simulink model "test_pfb_fft_full_full"  (R2013a)
settings: Solver=VariableStepDiscrete  StartTime=0.0  StopTime=1000  RelTol=1e-3
undefined symbols (not defined in the file): pfb_coeff_gen_calc
libraries: xbsIndex_r4 x21611, casper_library_misc x211, casper_library_delays x44, simulink x16, xbsReplacements_r4 x8, xps_library x1
(part 1 of 260)
settings Model: Creator=root  LastModifiedBy=root

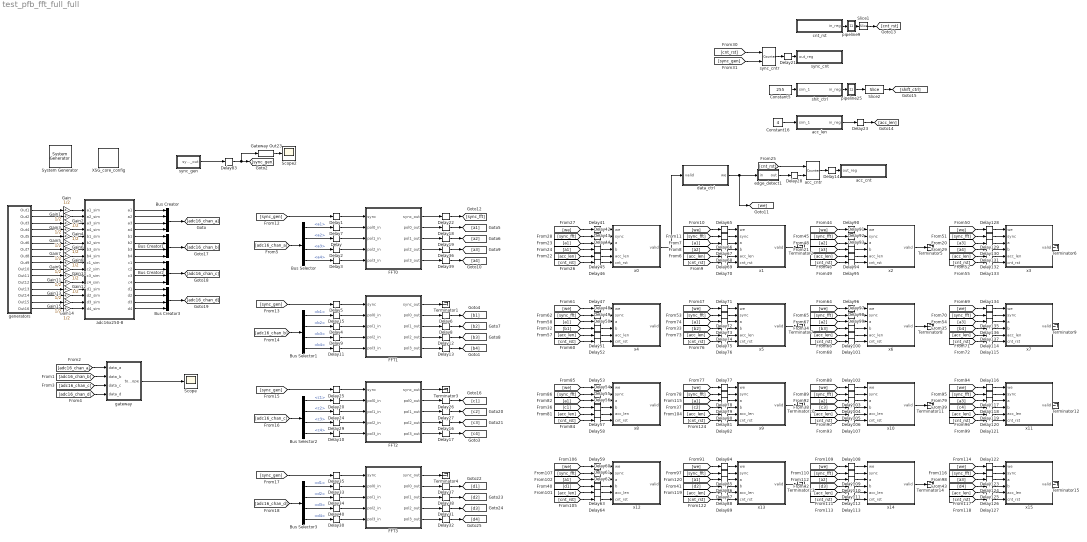
== system test_pfb_fft_full_full ==
  " System Generator" : xbsIndex_r4/ System Generator  Tag=genX  xilinxfamily=virtex6  part=xc6vsx475t  speed=-1  package=ff1759  synthesis_tool=XST  clock_wrapper=Clock Enables  directory=./test_pfb_fft_full_full/sysgen  proj_type=Project Navigator  Synth_file=XST Defaults  Impl_file=ISE Defaults  testbench=off  simulink_period=1  sysclk_period=5  dcm_input_clock_period=100  incr_netlist=off  trim_vbits=Everywhere in SubSystem  dbl_ovrd=According to Block Masks  core_generation=According to Block Masks  run_coregen=off  deprecated_control=off  eval_field=0  block_type=sysgen
  Bus Creator{,1..3} (4 alike) : BusCreator
  Bus Selector : BusSelector  OutputSignals=a1,a2,a3,a4
  Bus Selector1 : BusSelector  OutputSignals=b1,b2,b3,b4
  Bus Selector2 : BusSelector  OutputSignals=c1,c2,c3,c4
  Bus Selector3 : BusSelector  OutputSignals=d1,d2,d3,d4
  Constant16 : Constant  Value=4
  Constant5 : Constant  Value=255
  Delay{,1..5,7,9..11,14..15,20..21,23..25,29..30,33..35,40..137} (120 alike) : xbsIndex_r4/Delay  rst=off  infoeditControl=Selection of Reset will increase slice count due to use of real FFs and instead of SRLs  en=off  latency=2  dbl_ovrd=off  reg_retiming=on  block_type=delay
  Delay{6,8,12..13,16..19,22,26..28,31..32,36..39} (18 alike) : xbsIndex_r4/Delay  rst=off  infoeditControl=Selection of Reset will increase slice count due to use of real FFs and instead of SRLs  en=off  latency=1  dbl_ovrd=off  reg_retiming=on  block_type=delay
  FFT0 : Subsystem
  FFT1 : Subsystem
  FFT2 : Subsystem
  FFT3 : Subsystem
  Gain{,1..15} (16 alike) : Gain  Gain=1/2
  Gateway Out23 : xbsIndex_r4/Gateway Out  inherit_from_input=off  hdl_port=off  timing_constraint=None  locs_specified=off  LOCs={}  UseAsDAC=off  DACChannel='1'  block_type=gatewayout
      settings: infoedit="Gateway out block.  Converts Xilinx fixed point inputs into ouputs of type Simulink integer, double, or fixed point.<P><P>Hardware notes:  In hardware these blocks become top level output ports or are discarded, depending on how they are configured."  block_version=10.1.3
  Scope : Scope
  Scope2 : Scope
      settings: YMin=-1  YMax=1  MaxDataPoints=500000
  Slice1 : xbsIndex_r4/Slice  nbits=1  boolean_output=on  mode=Lower Bit Location + Width  bit1=0  base1=MSB of Input  bit0=0  base0=LSB of Input  dbl_ovrd=off  block_type=slice
      settings: infoedit="Extracts a given range of bits from each input sample and presents it at the output.  The output type is ordinarily unsigned with binary point at zero, but can be Boolean when the slice is one bit wide.<P><P>Hardware notes: In hardware this block costs nothing."  block_version=10.1.3
  Slice2 : xbsIndex_r4/Slice  nbits=12  boolean_output=off  mode=Lower Bit Location + Width  bit1=0  base1=MSB of Input  bit0=0  base0=LSB of Input  dbl_ovrd=off  block_type=slice
  Terminator{1..15} (15 alike) : Terminator
  XSG_core_config : xps_library/XSG_core_config  Tag=xps:xsg  hw_sys=ROACH2:sx475t  clk_src=adc0_clk  ROACH_clk_src=sys_clk  ROACH2_clk_src=adc0_clk  MKDIG_clk_src=sys_clk  clk_rate=200  sample_period=1  synthesis_tool=XST
  acc_cnt : Subsystem  Tag=xps:sw_reg  AncestorBlock=xps_library/software_register  mask(swreg){mode=one value, io_dir=To Processor, io_delay=0, sample_period=1, names=reg, bitwidths=32, arith_types=0, bin_pts=0, sim_port=off, show_format=off}
      mask prompts: io_dir "I/O direction"; io_delay "I/O delay"; sample_period "Sample period"; names "Name:"; bitwidths "Bitwidth:"; arith_types "Type (ufix=0, fix=1, bool=2):"; bin_pts "Binary pt:"; sim_port "Provide sim input/output?"; show_format "Print format string?"
      mask init:
      | swreg_init(gcb)
      settings: AttributesFormatString=1output
      mask TypeOptions mode: one value | fields of equal size | fields of arbitrary size
      mask Callback mode: swreg_cb(gcb);
      mask TypeOptions io_dir: To Processor | From Processor
      mask Callback io_dir:
      | mask_enables = get_param(gcb, 'MaskEnables');
      | mask_names = get_param(gcb, 'MaskNames');
      | if strcmp(get_param(gcb, 'io_dir'), 'To Processor'),
      |     mask_enables{ismember(mask_names, 'sample_period')} = 'off';
      | else
      |     mask_enables{ismember(mask_names, 'sample_period')} = 'on';
      | end
      | set_param(gcb, 'MaskEnables', mask_enables);
      mask Callback bitwidths:
      | swreg_maskcheck(gcb);
      | % blk = gcb;
      | % mode = get_param(blk, 'mode');
      | % current_widths = eval(get_param(blk, 'bitwidths'));
      | % if (strcmp(mode, 'one value') || strcmp(mode, 'fields of equal size')) && (length(current_widths) > 1),
      | %     error('Only one width may be specified for this mode.');
      | % elseif strcmp(mode, 'fields of arbitrary size')
      | %     current_names = textscan(strtrim(strrep(strrep(get_param(blk, 'names'), ']', ''), '[', '')), '%s');
      | %     current_names = current_names{1};
      | %     numios = length(current_names);
      | %     if length(current_widths) ~= numios,
      | %         error('%d field names specified, but %d widths?', numios, length(current_widths));
      | %     end
      | % end
      | % total_width = 0;
      | % for ctr = 1 : length(current_widths),
      | %     total_width = total_width + current_widths(ctr);
      | % end
      | % if total_width > 32,
      | %     error('Total width must be 32 or less, set to %d.', total_width);
      | % end
      mask Callback arith_types:
      | [numios, ~, ~, ~, current_types] = swreg_maskcheck(gcb);
      | % blk = gcb;
      | % mode = get_param(blk, 'mode');
      | % current_types = eval(get_param(blk, 'arith_types'));
      | % numios = length(current_types);
      | % if (strcmp(mode, 'one value') || strcmp(mode, 'fields of equal size')) && (length(current_types) > 1),
      | %     error('Only one type may be specified for this mode.');
      | % elseif strcmp(mode, 'fields of arbitrary size')
      | %     current_names = textscan(strtrim(strrep(strrep(get_param(blk, 'names'), ']', ''), '[', '')), '%s');
      | %     current_names = current_names{1};
      | %     numios = length(current_names);
      | %     if length(current_types) ~= numios,
      | %         error('%d field names specified, but %d types?', numios, length(current_types));
      | %     end
      | % end
      | problem = 0;
      | for ctr = 1 : numios,
      |     t = current_types(ctr);
      |     if (t < 0) || (t > 2),
      |         problem = t;
      |         ctr = numios + 1;
      |     end
      | end
      | if problem ~= 0,
      |     error('Type cannot be %i, must be 0, 1 or 2.', problem);
      | end
      mask Callback bin_pts:
      | swreg_maskcheck(gcb);
      | % blk = gcb;
      | % mode = get_param(blk, 'mode');
      | % current_bins = eval(get_param(blk, 'bin_pts'));
      | % if (strcmp(mode, 'one value') || strcmp(mode, 'fields of equal size')) && (length(current_bins) > 1),
      | %     error('Only one binary pt may be specified for this mode.');
      | % elseif strcmp(mode, 'fields of arbitrary size')
      | %     current_names = textscan(strtrim(strrep(strrep(get_param(blk, 'names'), ']', ''), '[', '')), '%s');
      | %     current_names = current_names{1};
      | %     numios = length(current_names);
      | %     if length(current_bins) ~= numios,
      | %         error('%d field names specified, but %d binary pts?', numios, length(current_bins));
      | %     end
      | % end
  acc_cntr : xbsIndex_r4/Counter  cnt_type=Free Running  cnt_to=Inf  operation=Up  start_count=0  cnt_by_val=1  arith_type=Unsigned  n_bits=32  bin_pt=0  load_pin=off  rst=on  en=on  explicit_period=on  period=1  dbl_ovrd=off  use_behavioral_HDL=off  implementation=Fabric  use_rpm=off  block_type=counter
  acc_len : Subsystem  Tag=xps:sw_reg  AncestorBlock=xps_library/software_register  mask(swreg){mode=one value, io_dir=From Processor, io_delay=0, sample_period=1, names=reg, bitwidths=32, arith_types=0, bin_pts=0, sim_port=on, show_format=off}
      mask prompts: io_dir "I/O direction"; io_delay "I/O delay"; sample_period "Sample period"; names "Name:"; bitwidths "Bitwidth:"; arith_types "Type (ufix=0, fix=1, bool=2):"; bin_pts "Binary pt:"; sim_port "Provide sim input/output?"; show_format "Print format string?"
      mask init:
      | swreg_init(gcb)
      settings: AttributesFormatString=1input
      mask TypeOptions mode: one value | fields of equal size | fields of arbitrary size
      mask Callback mode: swreg_cb(gcb);
      mask TypeOptions io_dir: To Processor | From Processor
      mask Callback io_dir:
      | mask_enables = get_param(gcb, 'MaskEnables');
      | mask_names = get_param(gcb, 'MaskNames');
      | if strcmp(get_param(gcb, 'io_dir'), 'To Processor'),
      |     mask_enables{ismember(mask_names, 'sample_period')} = 'off';
      | else
      |     mask_enables{ismember(mask_names, 'sample_period')} = 'on';
      | end
      | set_param(gcb, 'MaskEnables', mask_enables);
      mask Callback bitwidths:
      | swreg_maskcheck(gcb);
      | % blk = gcb;
      | % mode = get_param(blk, 'mode');
      | % current_widths = eval(get_param(blk, 'bitwidths'));
      | % if (strcmp(mode, 'one value') || strcmp(mode, 'fields of equal size')) && (length(current_widths) > 1),
      | %     error('Only one width may be specified for this mode.');
      | % elseif strcmp(mode, 'fields of arbitrary size')
      | %     current_names = textscan(strtrim(strrep(strrep(get_param(blk, 'names'), ']', ''), '[', '')), '%s');
      | %     current_names = current_names{1};
      | %     numios = length(current_names);
      | %     if length(current_widths) ~= numios,
      | %         error('%d field names specified, but %d widths?', numios, length(current_widths));
      | %     end
      | % end
      | % total_width = 0;
      | % for ctr = 1 : length(current_widths),
      | %     total_width = total_width + current_widths(ctr);
      | % end
      | % if total_width > 32,
      | %     error('Total width must be 32 or less, set to %d.', total_width);
      | % end
      mask Callback arith_types:
      | [numios, ~, ~, ~, current_types] = swreg_maskcheck(gcb);
      | % blk = gcb;
      | % mode = get_param(blk, 'mode');
      | % current_types = eval(get_param(blk, 'arith_types'));
      | % numios = length(current_types);
      | % if (strcmp(mode, 'one value') || strcmp(mode, 'fields of equal size')) && (length(current_types) > 1),
      | %     error('Only one type may be specified for this mode.');
      | % elseif strcmp(mode, 'fields of arbitrary size')
      | %     current_names = textscan(strtrim(strrep(strrep(get_param(blk, 'names'), ']', ''), '[', '')), '%s');
      | %     current_names = current_names{1};
      | %     numios = length(current_names);
      | %     if length(current_types) ~= numios,
      | %         error('%d field names specified, but %d types?', numios, length(current_types));
      | %     end
      | % end
      | problem = 0;
      | for ctr = 1 : numios,
      |     t = current_types(ctr);
      |     if (t < 0) || (t > 2),
      |         problem = t;
      |         ctr = numios + 1;
      |     end
      | end
      | if problem ~= 0,
      |     error('Type cannot be %i, must be 0, 1 or 2.', problem);
      | end
      mask Callback bin_pts:
      | swreg_maskcheck(gcb);
      | % blk = gcb;
      | % mode = get_param(blk, 'mode');
      | % current_bins = eval(get_param(blk, 'bin_pts'));
      | % if (strcmp(mode, 'one value') || strcmp(mode, 'fields of equal size')) && (length(current_bins) > 1),
      | %     error('Only one binary pt may be specified for this mode.');
      | % elseif strcmp(mode, 'fields of arbitrary size')
      | %     current_names = textscan(strtrim(strrep(strrep(get_param(blk, 'names'), ']', ''), '[', '')), '%s');
      | %     current_names = current_names{1};
      | %     numios = length(current_names);
      | %     if length(current_bins) ~= numios,
      | %         error('%d field names specified, but %d binary pts?', numios, length(current_bins));
      | %     end
      | % end
  adc16x250-8 : Subsystem  Tag=xps:adc16  AncestorBlock=xps_library/ADCs/adc16x250-8  mask(ADC16){board_count=1, roach2_rev=2, zdok_rev=2}
      mask prompts: board_count "Number of ADC16 Boards"; roach2_rev "ROACH2 Revision"; zdok_rev "ZDOK Pinout Revision"
      mask help: 16-input ADC. When using one ADC16 board, it must be in ZDOK0.
      mask init:
      | % Only board_count is needed by init function
      | adc16_init(gcb, ...
      |     'board_count', board_count);
      settings: AttributesFormatString=r2 rev%<roach2_rev>\nzdok rev%<zdok_rev>
      mask Help:
      | This is the CASPER "yellow block" for the CASPER ADC16 board.  Each ADC
      | board has four ADC chips.  Each ADC chip accepts four inputs.  The four
      | 8-bit outputs from each ADC chip are concatenated into a 32-bit signal.
      mask TypeOptions board_count: 1 | 2
      mask TypeOptions roach2_rev: 1 | 2
      mask TypeOptions zdok_rev: 1 | 2
  cnt_rst : Subsystem  Tag=xps:sw_reg  AncestorBlock=xps_library/software_register  mask(swreg){mode=one value, io_dir=From Processor, io_delay=0, sample_period=1, names=reg, bitwidths=32, arith_types=0, bin_pts=0, sim_port=off, show_format=off}
      mask prompts: io_dir "I/O direction"; io_delay "I/O delay"; sample_period "Sample period"; names "Name:"; bitwidths "Bitwidth:"; arith_types "Type (ufix=0, fix=1, bool=2):"; bin_pts "Binary pt:"; sim_port "Provide sim input/output?"; show_format "Print format string?"
      mask init:
      | swreg_init(gcb)
      settings: AttributesFormatString=1input
      mask TypeOptions mode: one value | fields of equal size | fields of arbitrary size
      mask Callback mode: swreg_cb(gcb);
      mask TypeOptions io_dir: To Processor | From Processor
      mask Callback io_dir:
      | mask_enables = get_param(gcb, 'MaskEnables');
      | mask_names = get_param(gcb, 'MaskNames');
      | if strcmp(get_param(gcb, 'io_dir'), 'To Processor'),
      |     mask_enables{ismember(mask_names, 'sample_period')} = 'off';
      | else
      |     mask_enables{ismember(mask_names, 'sample_period')} = 'on';
      | end
      | set_param(gcb, 'MaskEnables', mask_enables);
      mask Callback bitwidths:
      | swreg_maskcheck(gcb);
      | % blk = gcb;
      | % mode = get_param(blk, 'mode');
      | % current_widths = eval(get_param(blk, 'bitwidths'));
      | % if (strcmp(mode, 'one value') || strcmp(mode, 'fields of equal size')) && (length(current_widths) > 1),
      | %     error('Only one width may be specified for this mode.');
      | % elseif strcmp(mode, 'fields of arbitrary size')
      | %     current_names = textscan(strtrim(strrep(strrep(get_param(blk, 'names'), ']', ''), '[', '')), '%s');
      | %     current_names = current_names{1};
      | %     numios = length(current_names);
      | %     if length(current_widths) ~= numios,
      | %         error('%d field names specified, but %d widths?', numios, length(current_widths));
      | %     end
      | % end
      | % total_width = 0;
      | % for ctr = 1 : length(current_widths),
      | %     total_width = total_width + current_widths(ctr);
      | % end
      | % if total_width > 32,
      | %     error('Total width must be 32 or less, set to %d.', total_width);
      | % end
      mask Callback arith_types:
      | [numios, ~, ~, ~, current_types] = swreg_maskcheck(gcb);
      | % blk = gcb;
      | % mode = get_param(blk, 'mode');
      | % current_types = eval(get_param(blk, 'arith_types'));
      | % numios = length(current_types);
      | % if (strcmp(mode, 'one value') || strcmp(mode, 'fields of equal size')) && (length(current_types) > 1),
      | %     error('Only one type may be specified for this mode.');
      | % elseif strcmp(mode, 'fields of arbitrary size')
      | %     current_names = textscan(strtrim(strrep(strrep(get_param(blk, 'names'), ']', ''), '[', '')), '%s');
      | %     current_names = current_names{1};
      | %     numios = length(current_names);
      | %     if length(current_types) ~= numios,
      | %         error('%d field names specified, but %d types?', numios, length(current_types));
      | %     end
      | % end
      | problem = 0;
      | for ctr = 1 : numios,
      |     t = current_types(ctr);
      |     if (t < 0) || (t > 2),
      |         problem = t;
      |         ctr = numios + 1;
      |     end
      | end
      | if problem ~= 0,
      |     error('Type cannot be %i, must be 0, 1 or 2.', problem);
      | end
      mask Callback bin_pts:
      | swreg_maskcheck(gcb);
      | % blk = gcb;
      | % mode = get_param(blk, 'mode');
      | % current_bins = eval(get_param(blk, 'bin_pts'));
      | % if (strcmp(mode, 'one value') || strcmp(mode, 'fields of equal size')) && (length(current_bins) > 1),
      | %     error('Only one binary pt may be specified for this mode.');
      | % elseif strcmp(mode, 'fields of arbitrary size')
      | %     current_names = textscan(strtrim(strrep(strrep(get_param(blk, 'names'), ']', ''), '[', '')), '%s');
      | %     current_names = current_names{1};
      | %     numios = length(current_names);
      | %     if length(current_bins) ~= numios,
      | %         error('%d field names specified, but %d binary pts?', numios, length(current_bins));
      | %     end
      | % end
  data_ctrl : Subsystem  mask(data_ctrl){fft_size=2048, fft_outputs=4, accum=Single memory accumulator}
      mask prompts: fft_size "Number of channels (FFT size/2)"; fft_outputs "Number of parallel streams"; accum "Use accumulator?"
      mask init:
      | data_ctrl_init(gcb, 'fft_size', fft_size, ...
      |                     'fft_outputs', fft_outputs, ...
      |                     'accum', accum)
      mask TypeOptions accum: Dual memory accumulator | Single memory accumulator | No
      mask Display:
      | image(imread('calan.png'));
      | port_label('input',1,'valid');
      | 
      | if accum == 1
      |     port_label('output',1,'we0');
      |     port_label('output',2,'we1');
      | else
      |     port_label('output',1,'we');
      | end
  edge_detect1 : Subsystem  AncestorBlock=casper_library_misc/edge_detect  mask(edge_detect){edge=Falling, polarity=Active High, x_in=[0 0.08 0.08 0.16 0.16 0.24 0.24 0.32 0.32 0.4], y_in=[-1 -1  1  1  1  1  1  1  1  1], x_out=[0.6 0.68 0.68 0.76 0.76 0.84 0.84 0.92 0.92 1], y_out=[-1 -1  1  1 -1 -1 -1 -1 -1 -1]}
      mask prompts: edge "Edge Type"; polarity "Output Polarity"; x_in "Input X Positions"; y_in "Input Y Positions"; x_out "Output X Positions"; y_out "Output Y Positions"
      mask help: Output is active for one-cycle after each edge of the specified type (i.e. rising, falling or both).
      mask init:
      | edge_detect_init(gcb,...
      |     'edge',edge,...
      |     'polarity',polarity,...
      |     'x_in',x_in,...
      |     'x_out',x_out,...
      |     'y_in',y_in,...
      |     'y_out',y_out);
      | 
      | x_in_num=str2num(getfield(getfield(get_param(gcb,'UserData'),'parameters'),'x_in'));
      | x_out_num=str2num(getfield(getfield(get_param(gcb,'UserData'),'parameters'),'x_out'));
      | y_in_num=str2num(getfield(getfield(get_param(gcb,'UserData'),'parameters'),'y_in'));
      | y_out_num=str2num(getfield(getfield(get_param(gcb,'UserData'),'parameters'),'y_out'));
      settings: AttributesFormatString=|
      | falling edges
      | active high
      mask Help: Output is active for one-cycle after each edge of the specified type (i.e. rising, falling, or both).  Latency is zero.
      mask TypeOptions edge: Rising | Falling | Both
      mask TypeOptions polarity: Active High | Active Low
      mask Display:
      | plot(x_in_num,0.5+y_in_num*0.2);
      | plot(x_out_num,0.5+y_out_num*0.2);
  gateway : Subsystem
  generators : Subsystem
  pipeline25 : Subsystem  AncestorBlock=casper_library_delays/pipeline  mask(pipeline){latency=2}
      mask help:
      | An explicitly laid-out delay line to avoid mapping to SRL16s
      | for use in pipelining to help achieve timing closure.
      mask init:
      | pipeline_init(gcb,'latency',latency);
      settings: AttributesFormatString=Latency = %<latency>
      mask Display:
      | port_label('input', 1, 'd');
      | port_label('output', 1, 'q');
      | disp(['Z^-', num2str(latency)]);
  pipeline9 : Subsystem  AncestorBlock=casper_library_delays/pipeline  mask(pipeline){latency=2}
      mask help:
      | An explicitly laid-out delay line to avoid mapping to SRL16s
      | for use in pipelining to help achieve timing closure.
      mask init:
      | pipeline_init(gcb,'latency',latency);
      settings: AttributesFormatString=Latency = %<latency>
      mask Display:
      | port_label('input', 1, 'd');
      | port_label('output', 1, 'q');
      | disp(['Z^-', num2str(latency)]);
  shit_ctrl : Subsystem  Tag=xps:sw_reg  AncestorBlock=xps_library/software_register  mask(swreg){mode=one value, io_dir=From Processor, io_delay=0, sample_period=1, names=reg, bitwidths=32, arith_types=0, bin_pts=0, sim_port=on, show_format=off}
      mask prompts: io_dir "I/O direction"; io_delay "I/O delay"; sample_period "Sample period"; names "Name:"; bitwidths "Bitwidth:"; arith_types "Type (ufix=0, fix=1, bool=2):"; bin_pts "Binary pt:"; sim_port "Provide sim input/output?"; show_format "Print format string?"
      mask init:
      | swreg_init(gcb)
      settings: AttributesFormatString=1input
      mask TypeOptions mode: one value | fields of equal size | fields of arbitrary size
      mask Callback mode: swreg_cb(gcb);
      mask TypeOptions io_dir: To Processor | From Processor
      mask Callback io_dir:
      | mask_enables = get_param(gcb, 'MaskEnables');
      | mask_names = get_param(gcb, 'MaskNames');
      | if strcmp(get_param(gcb, 'io_dir'), 'To Processor'),
      |     mask_enables{ismember(mask_names, 'sample_period')} = 'off';
      | else
      |     mask_enables{ismember(mask_names, 'sample_period')} = 'on';
      | end
      | set_param(gcb, 'MaskEnables', mask_enables);
      mask Callback bitwidths:
      | swreg_maskcheck(gcb);
      | % blk = gcb;
      | % mode = get_param(blk, 'mode');
      | % current_widths = eval(get_param(blk, 'bitwidths'));
      | % if (strcmp(mode, 'one value') || strcmp(mode, 'fields of equal size')) && (length(current_widths) > 1),
      | %     error('Only one width may be specified for this mode.');
      | % elseif strcmp(mode, 'fields of arbitrary size')
      | %     current_names = textscan(strtrim(strrep(strrep(get_param(blk, 'names'), ']', ''), '[', '')), '%s');
      | %     current_names = current_names{1};
      | %     numios = length(current_names);
      | %     if length(current_widths) ~= numios,
      | %         error('%d field names specified, but %d widths?', numios, length(current_widths));
      | %     end
      | % end
      | % total_width = 0;
      | % for ctr = 1 : length(current_widths),
      | %     total_width = total_width + current_widths(ctr);
      | % end
      | % if total_width > 32,
      | %     error('Total width must be 32 or less, set to %d.', total_width);
      | % end
      mask Callback arith_types:
      | [numios, ~, ~, ~, current_types] = swreg_maskcheck(gcb);
      | % blk = gcb;
      | % mode = get_param(blk, 'mode');
      | % current_types = eval(get_param(blk, 'arith_types'));
      | % numios = length(current_types);
      | % if (strcmp(mode, 'one value') || strcmp(mode, 'fields of equal size')) && (length(current_types) > 1),
      | %     error('Only one type may be specified for this mode.');
      | % elseif strcmp(mode, 'fields of arbitrary size')
      | %     current_names = textscan(strtrim(strrep(strrep(get_param(blk, 'names'), ']', ''), '[', '')), '%s');
      | %     current_names = current_names{1};
      | %     numios = length(current_names);
      | %     if length(current_types) ~= numios,
      | %         error('%d field names specified, but %d types?', numios, length(current_types));
      | %     end
      | % end
      | problem = 0;
      | for ctr = 1 : numios,
      |     t = current_types(ctr);
      |     if (t < 0) || (t > 2),
      |         problem = t;
      |         ctr = numios + 1;
      |     end
      | end
      | if problem ~= 0,
      |     error('Type cannot be %i, must be 0, 1 or 2.', problem);
      | end
      mask Callback bin_pts:
      | swreg_maskcheck(gcb);
      | % blk = gcb;
      | % mode = get_param(blk, 'mode');
      | % current_bins = eval(get_param(blk, 'bin_pts'));
      | % if (strcmp(mode, 'one value') || strcmp(mode, 'fields of equal size')) && (length(current_bins) > 1),
      | %     error('Only one binary pt may be specified for this mode.');
      | % elseif strcmp(mode, 'fields of arbitrary size')
      | %     current_names = textscan(strtrim(strrep(strrep(get_param(blk, 'names'), ']', ''), '[', '')), '%s');
      | %     current_names = current_names{1};
      | %     numios = length(current_names);
      | %     if length(current_bins) ~= numios,
      | %         error('%d field names specified, but %d binary pts?', numios, length(current_bins));
      | %     end
      | % end
  sync_cnt : Subsystem  Tag=xps:sw_reg  AncestorBlock=xps_library/software_register  mask(swreg){mode=one value, io_dir=To Processor, io_delay=0, sample_period=1, names=reg, bitwidths=32, arith_types=0, bin_pts=0, sim_port=off, show_format=off}
      mask prompts: io_dir "I/O direction"; io_delay "I/O delay"; sample_period "Sample period"; names "Name:"; bitwidths "Bitwidth:"; arith_types "Type (ufix=0, fix=1, bool=2):"; bin_pts "Binary pt:"; sim_port "Provide sim input/output?"; show_format "Print format string?"
      mask init:
      | swreg_init(gcb)
      settings: AttributesFormatString=1output
      mask TypeOptions mode: one value | fields of equal size | fields of arbitrary size
      mask Callback mode: swreg_cb(gcb);
      mask TypeOptions io_dir: To Processor | From Processor
      mask Callback io_dir:
      | mask_enables = get_param(gcb, 'MaskEnables');
      | mask_names = get_param(gcb, 'MaskNames');
      | if strcmp(get_param(gcb, 'io_dir'), 'To Processor'),
      |     mask_enables{ismember(mask_names, 'sample_period')} = 'off';
      | else
      |     mask_enables{ismember(mask_names, 'sample_period')} = 'on';
      | end
      | set_param(gcb, 'MaskEnables', mask_enables);
      mask Callback bitwidths:
      | swreg_maskcheck(gcb);
      | % blk = gcb;
      | % mode = get_param(blk, 'mode');
      | % current_widths = eval(get_param(blk, 'bitwidths'));
      | % if (strcmp(mode, 'one value') || strcmp(mode, 'fields of equal size')) && (length(current_widths) > 1),
      | %     error('Only one width may be specified for this mode.');
      | % elseif strcmp(mode, 'fields of arbitrary size')
      | %     current_names = textscan(strtrim(strrep(strrep(get_param(blk, 'names'), ']', ''), '[', '')), '%s');
      | %     current_names = current_names{1};
      | %     numios = length(current_names);
      | %     if length(current_widths) ~= numios,
      | %         error('%d field names specified, but %d widths?', numios, length(current_widths));
      | %     end
      | % end
      | % total_width = 0;
      | % for ctr = 1 : length(current_widths),
      | %     total_width = total_width + current_widths(ctr);
      | % end
      | % if total_width > 32,
      | %     error('Total width must be 32 or less, set to %d.', total_width);
      | % end
      mask Callback arith_types:
      | [numios, ~, ~, ~, current_types] = swreg_maskcheck(gcb);
      | % blk = gcb;
      | % mode = get_param(blk, 'mode');
      | % current_types = eval(get_param(blk, 'arith_types'));
      | % numios = length(current_types);
      | % if (strcmp(mode, 'one value') || strcmp(mode, 'fields of equal size')) && (length(current_types) > 1),
      | %     error('Only one type may be specified for this mode.');
      | % elseif strcmp(mode, 'fields of arbitrary size')
      | %     current_names = textscan(strtrim(strrep(strrep(get_param(blk, 'names'), ']', ''), '[', '')), '%s');
      | %     current_names = current_names{1};
      | %     numios = length(current_names);
      | %     if length(current_types) ~= numios,
      | %         error('%d field names specified, but %d types?', numios, length(current_types));
      | %     end
      | % end
      | problem = 0;
      | for ctr = 1 : numios,
      |     t = current_types(ctr);
      |     if (t < 0) || (t > 2),
      |         problem = t;
      |         ctr = numios + 1;
      |     end
      | end
      | if problem ~= 0,
      |     error('Type cannot be %i, must be 0, 1 or 2.', problem);
      | end
      mask Callback bin_pts:
      | swreg_maskcheck(gcb);
      | % blk = gcb;
      | % mode = get_param(blk, 'mode');
      | % current_bins = eval(get_param(blk, 'bin_pts'));
      | % if (strcmp(mode, 'one value') || strcmp(mode, 'fields of equal size')) && (length(current_bins) > 1),
      | %     error('Only one binary pt may be specified for this mode.');
      | % elseif strcmp(mode, 'fields of arbitrary size')
      | %     current_names = textscan(strtrim(strrep(strrep(get_param(blk, 'names'), ']', ''), '[', '')), '%s');
      | %     current_names = current_names{1};
      | %     numios = length(current_names);
      | %     if length(current_bins) ~= numios,
      | %         error('%d field names specified, but %d binary pts?', numios, length(current_bins));
      | %     end
      | % end
  sync_cntr : xbsIndex_r4/Counter  cnt_type=Free Running  cnt_to=Inf  operation=Up  start_count=0  cnt_by_val=1  arith_type=Unsigned  n_bits=32  bin_pt=0  load_pin=off  rst=on  en=on  explicit_period=on  period=1  dbl_ovrd=off  use_behavioral_HDL=off  implementation=Fabric  use_rpm=off  block_type=counter
  sync_gen : Subsystem  AncestorBlock=casper_library_misc/sync_gen  mask(sync_gen){sim_acc_len=1, fft_size=256, fft_simult_inputs=1, pfb_fir_taps=4, reorder_vec=[2 2 2], scale=1}
      mask prompts: sim_acc_len "Simulation Accumulation Length"; fft_size "FFT Size"; fft_simult_inputs "Simultaneous Inputs (FFT)"; pfb_fir_taps "Taps in pfb_fir (use 1 if no pfb_fir)"; reorder_vec "Reorder Orders (e.g. [2 2 2])"
      mask help:
      | This block computes the sync period based on system parameters as
      | described in Casper memo.  Detailed descritions of the block's
      | variables can be found in the 'Block Documentation' section of
      | the CASPER wiki.
      mask init:
      | sync_gen_init(gcb, ...
      |     'sim_acc_len', sim_acc_len, ...
      |     'fft_size', fft_size, ...
      |     'fft_simult_inputs', fft_simult_inputs,...
      |     'pfb_fir_taps', pfb_fir_taps, ...
      |     'reorder_vec', reorder_vec, ...
      |     'scale', scale);
      settings: AttributesFormatString=sim_sync_period=2048
      mask Help:
      | SyncPeriod=LCM(reorder_vec)*(acc_num)*(pfb_fir_len)*(fft_size/fft_simult_inputs)
      | 
      | See CASPER sync pulse memo for more details.
  x0 : Subsystem
  x1 : Subsystem
  x10 : Subsystem
  x11 : Subsystem
  x12 : Subsystem
  x13 : Subsystem
  x14 : Subsystem
  x15 : Subsystem
  x2 : Subsystem
  x3 : Subsystem
  x4 : Subsystem
  x5 : Subsystem
  x6 : Subsystem
  x7 : Subsystem
  x8 : Subsystem
  x9 : Subsystem
  wires:
  adc16x250-8/3(a3) -> Bus Creator/3
  adc16x250-8/2(a2) -> Bus Creator/2
  adc16x250-8/4(a4) -> Bus Creator/4
  adc16x250-8/1(a1) -> Bus Creator/1
  Bus Creator/1 -> [adc16_chan_a]
  adc16x250-8/8(b4) -> Bus Creator1/4
  adc16x250-8/6(b2) -> Bus Creator1/2
  adc16x250-8/7(b3) -> Bus Creator1/3
  adc16x250-8/5(b1) -> Bus Creator1/1
  adc16x250-8/12(c4) -> Bus Creator2/4
  adc16x250-8/11(c3) -> Bus Creator2/3
  adc16x250-8/10(c2) -> Bus Creator2/2
  adc16x250-8/9(c1) -> Bus Creator2/1
  adc16x250-8/15(d3) -> Bus Creator3/3
  adc16x250-8/16(d4) -> Bus Creator3/4
  adc16x250-8/14(d2) -> Bus Creator3/2
  adc16x250-8/13(d1) -> Bus Creator3/1
  Bus Creator1/1 -> [adc16_chan_b]
  Bus Creator2/1 -> [adc16_chan_c]
  Bus Creator3/1 -> [adc16_chan_d]
  Gain/1 -> adc16x250-8/1(a1_sim)
  Gain1/1 -> adc16x250-8/2(a2_sim)
  Gain2/1 -> adc16x250-8/3(a3_sim)
  Gain3/1 -> adc16x250-8/4(a4_sim)
  Gain4/1 -> adc16x250-8/5(b1_sim)
  Gain5/1 -> adc16x250-8/6(b2_sim)
  Gain6/1 -> adc16x250-8/7(b3_sim)
  Gain7/1 -> adc16x250-8/8(b4_sim)
  Gain10/1 -> adc16x250-8/11(c3_sim)
  Gain8/1 -> adc16x250-8/9(c1_sim)
  Gain12/1 -> adc16x250-8/13(d1_sim)
  Gain15/1 -> adc16x250-8/16(d4_sim)
  Gain11/1 -> adc16x250-8/12(c4_sim)
  Gain14/1 -> adc16x250-8/15(d3_sim)
  Gain9/1 -> adc16x250-8/10(c2_sim)
  Gain13/1 -> adc16x250-8/14(d2_sim)
  gateway/1(to_scope) -> Scope/1
  [adc16_chan_a] -> gateway/1(data_a)
  [adc16_chan_b] -> gateway/2(data_b)
  [adc16_chan_c] -> gateway/3(data_c)
  [adc16_chan_d] -> gateway/4(data_d)
  [adc16_chan_a] -> Bus Selector/1
  generators/1(Out1) -> Gain/1
  generators/2(Out2) -> Gain1/1
  generators/3(Out3) -> Gain2/1
  generators/4(Out4) -> Gain3/1
  generators/5(Out5) -> Gain4/1
  generators/6(Out6) -> Gain5/1
  generators/7(Out7) -> Gain6/1
  generators/8(Out8) -> Gain7/1
  generators/9(Out9) -> Gain8/1
  generators/10(Out10) -> Gain9/1
  generators/11(Out11) -> Gain10/1
  generators/12(Out12) -> Gain11/1
  generators/13(Out13) -> Gain12/1
  generators/14(Out14) -> Gain13/1
  generators/15(Out15) -> Gain14/1
  generators/16(Out16) -> Gain15/1
  Delay22/1 -> [sync_fft]
  Delay18/1 -> [a1]
  Delay19/1 -> [a2]
  Delay36/1 -> [a3]
  Delay39/1 -> [a4]
  acc_cntr/1 -> Delay14/1
  Delay14/1 -> acc_cnt/1(out_reg)
  [cnt_rst] -> acc_cntr/1
  Delay28/1 -> acc_cntr/2
  edge_detect1/1(out) -> Delay28/1
  data_ctrl/1(we) -> [we], edge_detect1/1(in)
  x0/1(valid) -> data_ctrl/1(valid)
  [acc_len] -> Delay45/1
  [cnt_rst] -> Delay46/1
  [we] -> Delay41/1
  [sync_fft] -> Delay42/1
  [a1] -> Delay43/1
  [a1] -> Delay44/1
  cnt_rst/1(in_reg) -> pipeline9/1(d)
  pipeline9/1(q) -> Slice1/1
  Slice1/1 -> [cnt_rst]
  sync_cntr/1 -> Delay21/1
  Delay21/1 -> sync_cnt/1(out_reg)
  [cnt_rst] -> sync_cntr/1
  [sync_gen] -> sync_cntr/2
  Constant5/1 -> shit_ctrl/1(sim_1)
  pipeline25/1(q) -> Slice2/1
  shit_ctrl/1(in_reg) -> pipeline25/1(d)
  Slice2/1 -> [shift_ctrl]
  Constant16/1 -> acc_len/1(sim_1)
  Delay23/1 -> [acc_len]
  acc_len/1(in_reg) -> Delay23/1
  [acc_len] -> Delay69/1
  [cnt_rst] -> Delay70/1
  [we] -> Delay65/1
  [sync_fft] -> Delay66/1
  [a1] -> Delay67/1
  [a2] -> Delay68/1
  x1/1(valid) -> Terminator2/1
  sync_gen/1(sync_out) -> Delay83/1
  Gateway Out23/1 -> Scope2/1
  Delay83/1 -> [sync_gen], Gateway Out23/1
  Bus Selector/1 -> Delay7/1  "<a1>"
  Bus Selector/2 -> Delay/1  "<a2>"
  Bus Selector/3 -> Delay2/1  "<a3>"
  Bus Selector/4 -> Delay3/1  "<a4>"
  [sync_gen] -> Delay1/1
  Delay7/1 -> FFT0/2(pol0_in)
  Delay/1 -> FFT0/3(pol1_in)
  Delay2/1 -> FFT0/4(pol2_in)
  Delay3/1 -> FFT0/5(pol3_in)
  FFT0/1(sync_out) -> Delay22/1
  FFT0/2(pol0_out) -> Delay18/1
  FFT0/3(pol1_out) -> Delay19/1
  FFT0/4(pol2_out) -> Delay36/1
  FFT0/5(pol3_out) -> Delay39/1
  Delay1/1 -> FFT0/1(sync)
  [adc16_chan_b] -> Bus Selector1/1
  Delay6/1 -> [b1]
  Delay8/1 -> [b2]
  Delay12/1 -> [b3]
  Delay13/1 -> [b4]
  Bus Selector1/1 -> Delay15/1  "<b1>"
  Bus Selector1/2 -> Delay4/1  "<b2>"
  Bus Selector1/3 -> Delay9/1  "<b3>"
  Bus Selector1/4 -> Delay11/1  "<b4>"
  [sync_gen] -> Delay5/1
  Delay15/1 -> FFT1/2(pol0_in)
  Delay4/1 -> FFT1/3(pol1_in)
  Delay9/1 -> FFT1/4(pol2_in)
  Delay11/1 -> FFT1/5(pol3_in)
  FFT1/2(pol0_out) -> Delay6/1
  FFT1/3(pol1_out) -> Delay8/1
  FFT1/4(pol2_out) -> Delay12/1
  FFT1/5(pol3_out) -> Delay13/1
  Delay5/1 -> FFT1/1(sync)
  FFT1/1(sync_out) -> Terminator1/1
  [adc16_chan_c] -> Bus Selector2/1
  Delay26/1 -> [c1]
  Delay27/1 -> [c2]
  Delay16/1 -> [c3]
  Delay17/1 -> [c4]
  Bus Selector2/1 -> Delay20/1  "<c1>"
  Bus Selector2/2 -> Delay24/1  "<c2>"
  Bus Selector2/3 -> Delay29/1  "<c3>"
  Bus Selector2/4 -> Delay10/1  "<c4>"
  [sync_gen] -> Delay25/1
  Delay20/1 -> FFT2/2(pol0_in)
  Delay24/1 -> FFT2/3(pol1_in)
  Delay29/1 -> FFT2/4(pol2_in)
  Delay10/1 -> FFT2/5(pol3_in)
  FFT2/2(pol0_out) -> Delay26/1
  FFT2/3(pol1_out) -> Delay27/1
  FFT2/4(pol2_out) -> Delay16/1
  FFT2/5(pol3_out) -> Delay17/1
  Delay25/1 -> FFT2/1(sync)
  FFT2/1(sync_out) -> Terminator3/1
  [adc16_chan_d] -> Bus Selector3/1
  Delay37/1 -> [d1]
  Delay38/1 -> [d2]
  Delay31/1 -> [d3]
  Delay32/1 -> [d4]
  Bus Selector3/1 -> Delay33/1  "<d1>"
  Bus Selector3/2 -> Delay34/1  "<d2>"
  Bus Selector3/3 -> Delay40/1  "<d3>"
  Bus Selector3/4 -> Delay30/1  "<d4>"
  [sync_gen] -> Delay35/1
  Delay33/1 -> FFT3/2(pol0_in)
  Delay34/1 -> FFT3/3(pol1_in)
  Delay40/1 -> FFT3/4(pol2_in)
  Delay30/1 -> FFT3/5(pol3_in)
  FFT3/2(pol0_out) -> Delay37/1
  FFT3/3(pol1_out) -> Delay38/1
  FFT3/4(pol2_out) -> Delay31/1
  FFT3/5(pol3_out) -> Delay32/1
  Delay35/1 -> FFT3/1(sync)
  FFT3/1(sync_out) -> Terminator4/1
  [acc_len] -> Delay94/1
  [cnt_rst] -> Delay95/1
  [we] -> Delay90/1
  [sync_fft] -> Delay91/1
  x2/1(valid) -> Terminator5/1
  [acc_len] -> Delay132/1
  [cnt_rst] -> Delay133/1
  [we] -> Delay128/1
  [sync_fft] -> Delay129/1
  [a3] -> Delay130/1
  [a4] -> Delay131/1
  x3/1(valid) -> Terminator6/1
  [a2] -> Delay92/1
  [a3] -> Delay93/1
  [acc_len] -> Delay51/1
  [cnt_rst] -> Delay52/1
  [we] -> Delay47/1
  [sync_fft] -> Delay48/1
  [a1] -> Delay49/1
  [b1] -> Delay50/1
  [acc_len] -> Delay75/1
  [cnt_rst] -> Delay76/1
  [we] -> Delay71/1
  [sync_fft] -> Delay72/1
  [a1] -> Delay73/1
  [b2] -> Delay74/1
  x5/1(valid) -> Terminator7/1
  [acc_len] -> Delay100/1
  [cnt_rst] -> Delay101/1
  [we] -> Delay96/1
  [sync_fft] -> Delay97/1
  x6/1(valid) -> Terminator8/1
  [acc_len] -> Delay114/1
  [cnt_rst] -> Delay115/1
  [we] -> Delay134/1
  [sync_fft] -> Delay135/1
  [a3] -> Delay136/1
  [b4] -> Delay137/1
  x7/1(valid) -> Terminator9/1
  [a2] -> Delay98/1
  [b3] -> Delay99/1
  [acc_len] -> Delay57/1
  [cnt_rst] -> Delay58/1
  [we] -> Delay53/1
  [sync_fft] -> Delay54/1
  [a1] -> Delay55/1
  [c1] -> Delay56/1
  [acc_len] -> Delay81/1
  [cnt_rst] -> Delay82/1
  [we] -> Delay77/1
  [sync_fft] -> Delay78/1
  [a1] -> Delay79/1
  [c2] -> Delay80/1
  x9/1(valid) -> Terminator10/1
  [acc_len] -> Delay106/1
  [cnt_rst] -> Delay107/1
  [we] -> Delay102/1
  [sync_fft] -> Delay103/1
  x10/1(valid) -> Terminator11/1
  [acc_len] -> Delay120/1
  [cnt_rst] -> Delay121/1
  [we] -> Delay116/1
  [sync_fft] -> Delay117/1
  [a3] -> Delay118/1
  [c4] -> Delay119/1
  x11/1(valid) -> Terminator12/1
  [a2] -> Delay104/1
  [c3] -> Delay105/1
  [acc_len] -> Delay63/1
  [cnt_rst] -> Delay64/1
  [we] -> Delay59/1
  [sync_fft] -> Delay60/1
  [a1] -> Delay61/1
  [d1] -> Delay62/1
  [acc_len] -> Delay88/1
  [cnt_rst] -> Delay89/1
  [we] -> Delay84/1
  [sync_fft] -> Delay85/1
  [a1] -> Delay86/1
  [d2] -> Delay87/1
  x13/1(valid) -> Terminator13/1
  [acc_len] -> Delay112/1
  [cnt_rst] -> Delay113/1
  [we] -> Delay108/1
  [sync_fft] -> Delay109/1
  x14/1(valid) -> Terminator14/1
  [acc_len] -> Delay126/1
  [cnt_rst] -> Delay127/1
  [we] -> Delay122/1
  [sync_fft] -> Delay123/1
  [a3] -> Delay124/1
  [d4] -> Delay125/1
  x15/1(valid) -> Terminator15/1
  [a2] -> Delay110/1
  [d3] -> Delay111/1
  Delay41/1 -> x0/1(we)
  Delay42/1 -> x0/2(sync)
  Delay43/1 -> x0/3(a)
  Delay44/1 -> x0/4(b)
  Delay45/1 -> x0/5(acc_len)
  Delay46/1 -> x0/6(cnt_rst)
  Delay50/1 -> x4/4(b)
  Delay47/1 -> x4/1(we)
  Delay52/1 -> x4/6(cnt_rst)
  Delay48/1 -> x4/2(sync)
  Delay49/1 -> x4/3(a)
  Delay51/1 -> x4/5(acc_len)
  Delay63/1 -> x12/5(acc_len)
  Delay59/1 -> x12/1(we)
  Delay57/1 -> x8/5(acc_len)
  Delay60/1 -> x12/2(sync)
  Delay55/1 -> x8/3(a)
  Delay64/1 -> x12/6(cnt_rst)
  Delay56/1 -> x8/4(b)
  Delay58/1 -> x8/6(cnt_rst)
  Delay61/1 -> x12/3(a)
  Delay53/1 -> x8/1(we)
  Delay54/1 -> x8/2(sync)
  Delay62/1 -> x12/4(b)
  Delay89/1 -> x13/6(cnt_rst)
  Delay86/1 -> x13/3(a)
  Delay82/1 -> x9/6(cnt_rst)
  Delay84/1 -> x13/1(we)
  Delay71/1 -> x5/1(we)
  Delay69/1 -> x1/5(acc_len)
  Delay88/1 -> x13/5(acc_len)
  Delay78/1 -> x9/2(sync)
  Delay65/1 -> x1/1(we)
  Delay79/1 -> x9/3(a)
  Delay87/1 -> x13/4(b)
  Delay67/1 -> x1/3(a)
  Delay76/1 -> x5/6(cnt_rst)
  Delay73/1 -> x5/3(a)
  Delay68/1 -> x1/4(b)
  Delay72/1 -> x5/2(sync)
  Delay66/1 -> x1/2(sync)
  Delay70/1 -> x1/6(cnt_rst)
  Delay81/1 -> x9/5(acc_len)
  Delay75/1 -> x5/5(acc_len)
  Delay80/1 -> x9/4(b)
  Delay74/1 -> x5/4(b)
  Delay85/1 -> x13/2(sync)
  Delay77/1 -> x9/1(we)
  Delay101/1 -> x6/6(cnt_rst)
  Delay90/1 -> x2/1(we)
  Delay99/1 -> x6/4(b)
  Delay112/1 -> x14/5(acc_len)
  Delay110/1 -> x14/3(a)
  Delay92/1 -> x2/3(a)
  Delay109/1 -> x14/2(sync)
  Delay106/1 -> x10/5(acc_len)
  Delay91/1 -> x2/2(sync)
  Delay103/1 -> x10/2(sync)
  Delay97/1 -> x6/2(sync)
  Delay94/1 -> x2/5(acc_len)
  Delay100/1 -> x6/5(acc_len)
  Delay102/1 -> x10/1(we)
  Delay96/1 -> x6/1(we)
  Delay98/1 -> x6/3(a)
  Delay95/1 -> x2/6(cnt_rst)
  Delay108/1 -> x14/1(we)
  Delay107/1 -> x10/6(cnt_rst)
  Delay93/1 -> x2/4(b)
  Delay105/1 -> x10/4(b)
  Delay111/1 -> x14/4(b)
  Delay104/1 -> x10/3(a)
  Delay113/1 -> x14/6(cnt_rst)
  Delay115/1 -> x7/6(cnt_rst)
  Delay126/1 -> x15/5(acc_len)
  Delay134/1 -> x7/1(we)
  Delay127/1 -> x15/6(cnt_rst)
  Delay132/1 -> x3/5(acc_len)
  Delay125/1 -> x15/4(b)
  Delay133/1 -> x3/6(cnt_rst)
  Delay122/1 -> x15/1(we)
  Delay137/1 -> x7/4(b)
  Delay114/1 -> x7/5(acc_len)
  Delay130/1 -> x3/3(a)
  Delay120/1 -> x11/5(acc_len)
  Delay135/1 -> x7/2(sync)
  Delay123/1 -> x15/2(sync)
  Delay119/1 -> x11/4(b)
  Delay124/1 -> x15/3(a)
  Delay129/1 -> x3/2(sync)
  Delay136/1 -> x7/3(a)
  Delay121/1 -> x11/6(cnt_rst)
  Delay128/1 -> x3/1(we)
  Delay118/1 -> x11/3(a)
  Delay116/1 -> x11/1(we)
  Delay117/1 -> x11/2(sync)
  Delay131/1 -> x3/4(b)
